simulink model "V2x_TC_TrafficLights_cs"  (Simulink 7.6)
settings: Solver=ode5  SolverMode=SingleTasking  StartTime=0.0  StopTime=38  FixedStep=1/100  RelTol=1e-3
PreLoadFcn:
  | check_NUTS_defined
  | 
  | assignin('base',['model_closed_' bdroot],false);addpath('../includes_V2x');
InitFcn:
  | PreScanExperimentInitFcn(bdroot)
StopFcn:
  | PreScanExperimentStopFcn(bdroot);
  | 
undefined symbols (not defined in the file or in its folder): acceleration, actorID, actorType, DiscIntNeverNeededParam, GPS_alt, GPS_lat, GPS_long, intmax, lightBarInUse, messageID, Newton, publicActorType, Raphson, rotZ, sigbuilder_block, sireneInUse, trafficLightPriority, turnAdvice, velocity, x_ini_VRML, y_ini_VRML, yawRate, z_ini_VRML
libraries: simulink x172, PreScanSimulinkLibrary x20, PreScanUsefulBlocks x11, simulink_extras x9, simulink_need_slupdate x1
(part 4 of 27)

== system V2x_TC_TrafficLights_cs/STATE_Sphere_1 ==
  State : Inport  SampleTime=TimeStep
  Acceleration : Subsystem  mask(ToSensorWorld){PortName=Acceleration_Sphere_1, PortIndex=1630, PortWidth=3, TimeStep=1/100}
      mask prompts: PortName "Port Name [-]"; PortIndex "Index [-]"; PortWidth "Port Dimensions [-]"; TimeStep "Upload Period [s]"
      mask help: Sends signal(s) from Simulink to the Sensor World
      mask Help:
      | Sends signal(s) from Simulink to the Sensor World.
      | 
      | - Port Name [-]: specifies the name of the output terminal of the Simulink block.
      | - Index [-]: specifies the start position in the output vector sent to the Sensor World
      | - Port dimensions [-]: specifies the width (number of scalars) of the output port
      | - Upload period [s]: specifies the upload period (in seconds) of the port.
      mask Display: port_label('input', 1, PortName)
  Demux : Demux  Outputs=5
  Position : Subsystem  mask(ToSensorWorld){PortName=Position_Sphere_1, PortIndex=1624, PortWidth=3, TimeStep=1/100}
      mask prompts: PortName "Port Name [-]"; PortIndex "Index [-]"; PortWidth "Port Dimensions [-]"; TimeStep "Upload Period [s]"
      mask help: Sends signal(s) from Simulink to the Sensor World
      mask Help:
      | Sends signal(s) from Simulink to the Sensor World.
      | 
      | - Port Name [-]: specifies the name of the output terminal of the Simulink block.
      | - Index [-]: specifies the start position in the output vector sent to the Sensor World
      | - Port dimensions [-]: specifies the width (number of scalars) of the output port
      | - Upload period [s]: specifies the upload period (in seconds) of the port.
      mask Display: port_label('input', 1, PortName)
  RPY : Subsystem  mask(ToSensorWorld){PortName=RPY_Sphere_1, PortIndex=1633, PortWidth=3, TimeStep=1/100}
      mask prompts: PortName "Port Name [-]"; PortIndex "Index [-]"; PortWidth "Port Dimensions [-]"; TimeStep "Upload Period [s]"
      mask help: Sends signal(s) from Simulink to the Sensor World
      mask Help:
      | Sends signal(s) from Simulink to the Sensor World.
      | 
      | - Port Name [-]: specifies the name of the output terminal of the Simulink block.
      | - Index [-]: specifies the start position in the output vector sent to the Sensor World
      | - Port dimensions [-]: specifies the width (number of scalars) of the output port
      | - Upload period [s]: specifies the upload period (in seconds) of the port.
      mask Display: port_label('input', 1, PortName)
  Velocity : Subsystem  mask(ToSensorWorld){PortName=Velocity_Sphere_1, PortIndex=1627, PortWidth=3, TimeStep=1/100}
      mask prompts: PortName "Port Name [-]"; PortIndex "Index [-]"; PortWidth "Port Dimensions [-]"; TimeStep "Upload Period [s]"
      mask help: Sends signal(s) from Simulink to the Sensor World
      mask Help:
      | Sends signal(s) from Simulink to the Sensor World.
      | 
      | - Port Name [-]: specifies the name of the output terminal of the Simulink block.
      | - Index [-]: specifies the start position in the output vector sent to the Sensor World
      | - Port dimensions [-]: specifies the width (number of scalars) of the output port
      | - Upload period [s]: specifies the upload period (in seconds) of the port.
      mask Display: port_label('input', 1, PortName)
  iniBODYAngles wrtVEH [rad] : Constant  Value=[Roll0 Pitch0 0]  SampleTime=TimeStep
  iniVEHAngles wrtGLOB [rad] : Constant  Value=[0 0 Yaw0]  SampleTime=TimeStep
  iniVEHposition wrtGLOB [m] : Constant  Value=[x0 y0 z0]  SampleTime=TimeStep
  state2FullState : PreScanSimulinkLibrary/state2FullState  SystemSampleTime=-1
  velRPY : Subsystem  mask(ToSensorWorld){PortName=velRPY_Sphere_1, PortIndex=1636, PortWidth=3, TimeStep=1/100}
      mask prompts: PortName "Port Name [-]"; PortIndex "Index [-]"; PortWidth "Port Dimensions [-]"; TimeStep "Upload Period [s]"
      mask help: Sends signal(s) from Simulink to the Sensor World
      mask Help:
      | Sends signal(s) from Simulink to the Sensor World.
      | 
      | - Port Name [-]: specifies the name of the output terminal of the Simulink block.
      | - Index [-]: specifies the start position in the output vector sent to the Sensor World
      | - Port dimensions [-]: specifies the width (number of scalars) of the output port
      | - Upload period [s]: specifies the upload period (in seconds) of the port.
      mask Display: port_label('input', 1, PortName)
  wires:
  Demux/5 -> velRPY/1(Y)
  Demux/4 -> RPY/1(Y)
  Demux/3 -> Acceleration/1(Y)
  Demux/2 -> Velocity/1(Y)
  Demux/1 -> Position/1(Y)
  State/1 -> state2FullState/1
  state2FullState/1 -> Demux/1
  iniVEHposition wrtGLOB [m]/1 -> state2FullState/2
  iniBODYAngles wrtVEH [rad]/1 -> state2FullState/3
  iniVEHAngles wrtGLOB [rad]/1 -> state2FullState/4

== system V2x_TC_TrafficLights_cs/SetActuators ==
  Bus Creator : BusCreator  Inputs=1
  Data Store Read : DataStoreRead  DataStoreName=SimCoreActuators  SampleTime=TimeStep
  SendActuators : M-S-Function  FunctionName=SendActuatorsBlock  Parameters=TimeStep NrOfActuators
  dummy : Outport
  tag scope: SimCoreInfo=global
  wires:
  Bus Creator/1 -> [SimCoreInfo]  "SimCore"
  SendActuators/2 -> Bus Creator/1  "ComputationTime[s]"
  SendActuators/1 -> dummy/1
  Data Store Read/1 -> SendActuators/1

== system V2x_TC_TrafficLights_cs/SimCoreInfo ==
  Bus Selector : BusSelector  OutputSignals=ComputationTime[s]
  Time : Scope
      settings: TimeRange=10  YMin=0  YMax=0.5  SaveName=ComputationTime
  wires:
  Bus Selector/1 -> Time/1  "<ComputationTime[s]>"
  [SimCoreInfo] -> Bus Selector/1

== system V2x_TC_TrafficLights_cs/Sphere_1 ==
  SELF_Sphere_1 : Inport  Tag=SELFInport
  Dynamics_Empty : Subsystem  Tag=Dynamics_Empty
      mask Display: port_label('output', 1, 'State');text(0,0,' Empty\n')
  Gain : Gain  Gain=[1 1 1 0 0 0 0 0 1]
  SELF_Demux : Subsystem  Tag=DemuxSELF
      settings: DeleteFcn=PreScanBlockDeleteFcn;
      mask Type: PreScan Demux Block:
      mask Display:
      | port_label('output',1,'x [m]')
      | port_label('output',2,'y [m]')
      | port_label('output',3,'z [m]','texmode','on')
      | port_label('output',4,'Rotx [deg]','texmode','on')
      | port_label('output',5,'Roty [deg]','texmode','on')
      | port_label('output',6,'Rotz [deg]','texmode','on')
      | port_label('output',7,'GPS lat [deg]','texmode','on')
      | port_label('output',8,'GPS long [deg]','texmode','on')
      | port_label('output',9,'GPS alt [m]','texmode','on')
      | port_label('output',10,'Velocity [m/s]')
      | port_label('output',11,'Heading [deg]')
      | port_label('output',12,'Yaw rate [deg/s]')
  Terminator_{1..13} (13 alike) : Terminator
  STATE_Sphere_1 : Outport  Tag=StateOutport
  wires:
  [State] -> Gain/1
  SELF_Sphere_1/1 -> SELF_Demux/1(SELF)
  Dynamics_Empty/1(State) -> Terminator_13/1
  Gain/1 -> STATE_Sphere_1/1
  SELF_Demux/1(x [m]) -> Terminator_1/1
  SELF_Demux/2(y [m]) -> Terminator_2/1
  SELF_Demux/3(z [m]) -> Terminator_3/1
  SELF_Demux/4(rotx [deg]) -> Terminator_4/1
  SELF_Demux/5(roty [deg]) -> Terminator_5/1
  SELF_Demux/6(rotz [deg]) -> Terminator_6/1
  SELF_Demux/7(GPS lat [deg]) -> Terminator_7/1
  SELF_Demux/8(GPS long [deg]) -> Terminator_8/1
  SELF_Demux/9(GPS alt [m]) -> Terminator_9/1
  SELF_Demux/10(velocity [m/s]) -> Terminator_10/1
  SELF_Demux/11(heading [deg]) -> Terminator_11/1
  SELF_Demux/12(yaw rate [deg/s]) -> Terminator_12/1

== system V2x_TC_TrafficLights_cs/TrafficLightRoadSideNL_1 ==
  Antenna_Tx_3_BusAssignment : BusAssignment  Tag=BusAntennaT  AssignedSignals=warning,messageID,actorID,actorType,trafficLightPriority,GPS_lat,GPS_long,GPS_alt,rotZ
  Antenna_Tx_3_Mux : Subsystem  Tag=MuxAntennaT  mask(PreScan Mux Block:){Channel=1, TimeStep=1/100}
      mask prompts: TimeStep "Time Step [s]"
      settings: DeleteFcn=PreScanBlockDeleteFcn;
      mask Display:
      | fprintf('Channel: %d',Channel)
      | port_label('input', 1, 'Warning signal ID')
      | port_label('input', 2, 'Maneuvre ID')
      | port_label('input', 3, 'Vehicle Type')
      | port_label('input', 4, 'Braking Capacity')
      | port_label('input', 5, 'Vehicle Size')
      | port_label('input', 6, 'spare')
  CAM generation time step : Constant  Value=0.1
  CAM repetition time step : Constant  Value=0.05
  Cartesian to GPS : Subsystem
  Ground_{1..5} (5 alike) : Ground
  Infrastructure CAM generator : Subsystem
  VISUCasingLights_Mux : Subsystem  Tag=MuxCasingLights
      settings: DeleteFcn=PreScanBlockDeleteFcn;
      mask Type: PreScan Mux Block:
  X : Constant  Value=112.02973175048828
  Y : Constant  Value=9.0402154922485352
  Z : Constant  Value=0
  actorID : Constant  Value=13
  actorType : Constant  Value=15
  different coordinate system conversion : Bias  Bias=-90
  lights : Subsystem
  messageID : Constant  Value=900
  rotZ : Constant  Value=270
  warning : Constant  Value=0
  Antenna_Tx_3 : Outport  Tag=AntennaTOutport
  VISUCasingLights_TrafficLightRoadSideNL_1 : Outport  Tag=VisuCasingLightsOutport  Port=2  SampleTime=1/100
  wires:
  CAM generation time step/1 -> Infrastructure CAM generator/1(CAM gen time step)
  CAM repetition time step/1 -> Infrastructure CAM generator/2(CAM rep time step)
  warning/1 -> Infrastructure CAM generator/3(warning)
  messageID/1 -> Infrastructure CAM generator/4(messageID)
  actorID/1 -> Infrastructure CAM generator/5(actorID)
  actorType/1 -> Infrastructure CAM generator/6(actorType)
  lights/1(R) -> Infrastructure CAM generator/7(Red light), VISUCasingLights_Mux/1(Red [-])
  lights/2(O) -> Infrastructure CAM generator/8(Orange light), VISUCasingLights_Mux/2(Orange [-])
  lights/3(G) -> Infrastructure CAM generator/9(Green light), VISUCasingLights_Mux/3(Green [-])
  rotZ/1 -> different coordinate system conversion/1
  Cartesian to GPS/1(lat) -> Infrastructure CAM generator/10(GPS_lat)
  Cartesian to GPS/2(long) -> Infrastructure CAM generator/11(GPS_long)
  Cartesian to GPS/3(alt) -> Infrastructure CAM generator/12(GPS_alt)
  X/1 -> Cartesian to GPS/1(X)
  Y/1 -> Cartesian to GPS/2(Y)
  Z/1 -> Cartesian to GPS/3(Z)
  different coordinate system conversion/1 -> Infrastructure CAM generator/13(rotZ)
  Antenna_Tx_3_Mux/2(Out2) -> [Antenna_Tx_3]
  [Antenna_Tx_3] -> Antenna_Tx_3_BusAssignment/1
  Antenna_Tx_3_BusAssignment/1 -> Antenna_Tx_3_Mux/6(spare)
  Antenna_Tx_3_Mux/1(Out1) -> Antenna_Tx_3/1
  VISUCasingLights_Mux/1(CasingLights) -> VISUCasingLights_TrafficLightRoadSideNL_1/1
  Infrastructure CAM generator/9(CAM_rotZ) -> Antenna_Tx_3_BusAssignment/10
  Infrastructure CAM generator/8(CAM_GPSalt) -> Antenna_Tx_3_BusAssignment/9
  Infrastructure CAM generator/7(CAM_GPSlong) -> Antenna_Tx_3_BusAssignment/8
  Infrastructure CAM generator/6(CAM_GPSlat) -> Antenna_Tx_3_BusAssignment/7
  Infrastructure CAM generator/5(CAM_trafficLightPriority) -> Antenna_Tx_3_BusAssignment/6
  Infrastructure CAM generator/4(CAM_vehicleType) -> Antenna_Tx_3_BusAssignment/5
  Infrastructure CAM generator/3(CAM_vehicleID) -> Antenna_Tx_3_BusAssignment/4
  Infrastructure CAM generator/2(CAM_messageID) -> Antenna_Tx_3_BusAssignment/3
  Infrastructure CAM generator/1(CAM_warning) -> Antenna_Tx_3_BusAssignment/2
  Ground_1/1 -> Antenna_Tx_3_Mux/1(warning_signal_id)
  Ground_2/1 -> Antenna_Tx_3_Mux/2(maneuvre_id)
  Ground_3/1 -> Antenna_Tx_3_Mux/3(vehicle_type)
  Ground_4/1 -> Antenna_Tx_3_Mux/4(brakingcapacity)
  Ground_5/1 -> Antenna_Tx_3_Mux/5(vehicle_size)

== system V2x_TC_TrafficLights_cs/TrafficLightRoadSideNL_2 ==
  Antenna_Tx_5_BusAssignment : BusAssignment  Tag=BusAntennaT  AssignedSignals=warning,messageID,actorID,actorType,trafficLightPriority,GPS_lat,GPS_long,GPS_alt,rotZ
  Antenna_Tx_5_Mux : Subsystem  Tag=MuxAntennaT  mask(PreScan Mux Block:){Channel=1, TimeStep=1/100}
      mask prompts: TimeStep "Time Step [s]"
      settings: DeleteFcn=PreScanBlockDeleteFcn;
      mask Display:
      | fprintf('Channel: %d',Channel)
      | port_label('input', 1, 'Warning signal ID')
      | port_label('input', 2, 'Maneuvre ID')
      | port_label('input', 3, 'Vehicle Type')
      | port_label('input', 4, 'Braking Capacity')
      | port_label('input', 5, 'Vehicle Size')
      | port_label('input', 6, 'spare')
  CAM generation time step : Constant  Value=0.1
  CAM repetition time step : Constant  Value=0.05
  Cartesian to GPS : Subsystem
  Ground_{1..5} (5 alike) : Ground
  Infrastructure CAM generator : Subsystem
  VISUCasingLights_Mux : Subsystem  Tag=MuxCasingLights
      settings: DeleteFcn=PreScanBlockDeleteFcn;
      mask Type: PreScan Mux Block:
  X : Constant  Value=123.47191619873047
  Y : Constant  Value=17.9848575592041
  Z : Constant  Value=0
  actorID : Constant  Value=16
  actorType : Constant  Value=15
  different coordinate system conversion : Bias  Bias=-90
  lights : Subsystem
  messageID : Constant  Value=900
  rotZ : Constant  Value=90
  warning : Constant  Value=0
  Antenna_Tx_5 : Outport  Tag=AntennaTOutport
  VISUCasingLights_TrafficLightRoadSideNL_2 : Outport  Tag=VisuCasingLightsOutport  Port=2  SampleTime=1/100
  wires:
  CAM generation time step/1 -> Infrastructure CAM generator/1(CAM gen time step)
  CAM repetition time step/1 -> Infrastructure CAM generator/2(CAM rep time step)
  warning/1 -> Infrastructure CAM generator/3(warning)
  messageID/1 -> Infrastructure CAM generator/4(messageID)
  actorID/1 -> Infrastructure CAM generator/5(actorID)
  actorType/1 -> Infrastructure CAM generator/6(actorType)
  lights/1(R) -> Infrastructure CAM generator/7(Red light), VISUCasingLights_Mux/1(Red [-])
  lights/2(O) -> Infrastructure CAM generator/8(Orange light), VISUCasingLights_Mux/2(Orange [-])
  lights/3(G) -> Infrastructure CAM generator/9(Green light), VISUCasingLights_Mux/3(Green [-])
  rotZ/1 -> different coordinate system conversion/1
  Cartesian to GPS/1(lat) -> Infrastructure CAM generator/10(GPS_lat)
  Cartesian to GPS/2(long) -> Infrastructure CAM generator/11(GPS_long)
  Cartesian to GPS/3(alt) -> Infrastructure CAM generator/12(GPS_alt)
  X/1 -> Cartesian to GPS/1(X)
  Y/1 -> Cartesian to GPS/2(Y)
  Z/1 -> Cartesian to GPS/3(Z)
  different coordinate system conversion/1 -> Infrastructure CAM generator/13(rotZ)
  Antenna_Tx_5_Mux/2(Out2) -> [Antenna_Tx_5]
  [Antenna_Tx_5] -> Antenna_Tx_5_BusAssignment/1
  Antenna_Tx_5_BusAssignment/1 -> Antenna_Tx_5_Mux/6(spare)
  Antenna_Tx_5_Mux/1(Out1) -> Antenna_Tx_5/1
  VISUCasingLights_Mux/1(CasingLights) -> VISUCasingLights_TrafficLightRoadSideNL_2/1
  Infrastructure CAM generator/9(CAM_rotZ) -> Antenna_Tx_5_BusAssignment/10
  Infrastructure CAM generator/8(CAM_GPSalt) -> Antenna_Tx_5_BusAssignment/9
  Infrastructure CAM generator/7(CAM_GPSlong) -> Antenna_Tx_5_BusAssignment/8
  Infrastructure CAM generator/6(CAM_GPSlat) -> Antenna_Tx_5_BusAssignment/7
  Infrastructure CAM generator/5(CAM_trafficLightPriority) -> Antenna_Tx_5_BusAssignment/6
  Infrastructure CAM generator/4(CAM_vehicleType) -> Antenna_Tx_5_BusAssignment/5
  Infrastructure CAM generator/3(CAM_vehicleID) -> Antenna_Tx_5_BusAssignment/4
  Infrastructure CAM generator/2(CAM_messageID) -> Antenna_Tx_5_BusAssignment/3
  Infrastructure CAM generator/1(CAM_warning) -> Antenna_Tx_5_BusAssignment/2
  Ground_1/1 -> Antenna_Tx_5_Mux/1(warning_signal_id)
  Ground_2/1 -> Antenna_Tx_5_Mux/2(maneuvre_id)
  Ground_3/1 -> Antenna_Tx_5_Mux/3(vehicle_type)
  Ground_4/1 -> Antenna_Tx_5_Mux/4(brakingcapacity)
  Ground_5/1 -> Antenna_Tx_5_Mux/5(vehicle_size)

== system V2x_TC_TrafficLights_cs/TrafficLightRoadSideNL_3 ==
  Antenna_Tx_6_BusAssignment : BusAssignment  Tag=BusAntennaT  AssignedSignals=warning,messageID,actorID,actorType,trafficLightPriority,GPS_lat,GPS_long,GPS_alt,rotZ
  Antenna_Tx_6_Mux : Subsystem  Tag=MuxAntennaT  mask(PreScan Mux Block:){Channel=1, TimeStep=1/100}
      mask prompts: TimeStep "Time Step [s]"
      settings: DeleteFcn=PreScanBlockDeleteFcn;
      mask Display:
      | fprintf('Channel: %d',Channel)
      | port_label('input', 1, 'Warning signal ID')
      | port_label('input', 2, 'Maneuvre ID')
      | port_label('input', 3, 'Vehicle Type')
      | port_label('input', 4, 'Braking Capacity')
      | port_label('input', 5, 'Vehicle Size')
      | port_label('input', 6, 'spare')
  CAM generation time step : Constant  Value=0.1
  CAM repetition time step : Constant  Value=0.05
  Cartesian to GPS : Subsystem
  Ground_{1..5} (5 alike) : Ground
  Infrastructure CAM generator : Subsystem
  VISUCasingLights_Mux : Subsystem  Tag=MuxCasingLights
      settings: DeleteFcn=PreScanBlockDeleteFcn;
      mask Type: PreScan Mux Block:
  X : Constant  Value=122.22503662109375
  Y : Constant  Value=7.7345576286315918
  Z : Constant  Value=0
  actorID : Constant  Value=14
  actorType : Constant  Value=15
  different coordinate system conversion : Bias  Bias=-90
  lights : Subsystem
  messageID : Constant  Value=900
  rotZ : Constant  Value=0
  warning : Constant  Value=0
  Antenna_Tx_6 : Outport  Tag=AntennaTOutport
  VISUCasingLights_TrafficLightRoadSideNL_3 : Outport  Tag=VisuCasingLightsOutport  Port=2  SampleTime=1/100
  wires:
  CAM generation time step/1 -> Infrastructure CAM generator/1(CAM gen time step)
  CAM repetition time step/1 -> Infrastructure CAM generator/2(CAM rep time step)
  warning/1 -> Infrastructure CAM generator/3(warning)
  messageID/1 -> Infrastructure CAM generator/4(messageID)
  actorID/1 -> Infrastructure CAM generator/5(actorID)
  actorType/1 -> Infrastructure CAM generator/6(actorType)
  rotZ/1 -> different coordinate system conversion/1
  Cartesian to GPS/1(lat) -> Infrastructure CAM generator/10(GPS_lat)
  Cartesian to GPS/2(long) -> Infrastructure CAM generator/11(GPS_long)
  Cartesian to GPS/3(alt) -> Infrastructure CAM generator/12(GPS_alt)
  X/1 -> Cartesian to GPS/1(X)
  Y/1 -> Cartesian to GPS/2(Y)
  Z/1 -> Cartesian to GPS/3(Z)
  lights/1(R) -> Infrastructure CAM generator/7(Red light), VISUCasingLights_Mux/1(Red [-])
  lights/2(O) -> Infrastructure CAM generator/8(Orange light), VISUCasingLights_Mux/2(Orange [-])
  lights/3(G) -> Infrastructure CAM generator/9(Green light), VISUCasingLights_Mux/3(Green [-])
  different coordinate system conversion/1 -> Infrastructure CAM generator/13(rotZ)
  Antenna_Tx_6_Mux/2(Out2) -> [Antenna_Tx_6]
  [Antenna_Tx_6] -> Antenna_Tx_6_BusAssignment/1
  Antenna_Tx_6_BusAssignment/1 -> Antenna_Tx_6_Mux/6(spare)
  Antenna_Tx_6_Mux/1(Out1) -> Antenna_Tx_6/1
  VISUCasingLights_Mux/1(CasingLights) -> VISUCasingLights_TrafficLightRoadSideNL_3/1
  Infrastructure CAM generator/9(CAM_rotZ) -> Antenna_Tx_6_BusAssignment/10
  Infrastructure CAM generator/8(CAM_GPSalt) -> Antenna_Tx_6_BusAssignment/9
  Infrastructure CAM generator/7(CAM_GPSlong) -> Antenna_Tx_6_BusAssignment/8
  Infrastructure CAM generator/6(CAM_GPSlat) -> Antenna_Tx_6_BusAssignment/7
  Infrastructure CAM generator/5(CAM_trafficLightPriority) -> Antenna_Tx_6_BusAssignment/6
  Infrastructure CAM generator/4(CAM_vehicleType) -> Antenna_Tx_6_BusAssignment/5
  Infrastructure CAM generator/3(CAM_vehicleID) -> Antenna_Tx_6_BusAssignment/4
  Infrastructure CAM generator/2(CAM_messageID) -> Antenna_Tx_6_BusAssignment/3
  Infrastructure CAM generator/1(CAM_warning) -> Antenna_Tx_6_BusAssignment/2
  Ground_1/1 -> Antenna_Tx_6_Mux/1(warning_signal_id)
  Ground_2/1 -> Antenna_Tx_6_Mux/2(maneuvre_id)
  Ground_3/1 -> Antenna_Tx_6_Mux/3(vehicle_type)
  Ground_4/1 -> Antenna_Tx_6_Mux/4(brakingcapacity)
  Ground_5/1 -> Antenna_Tx_6_Mux/5(vehicle_size)

== system V2x_TC_TrafficLights_cs/TrafficLightRoadSideNL_4 ==
  Antenna_Tx_4_BusAssignment : BusAssignment  Tag=BusAntennaT  AssignedSignals=warning,messageID,actorID,actorType,trafficLightPriority,GPS_lat,GPS_long,GPS_alt,rotZ
  Antenna_Tx_4_Mux : Subsystem  Tag=MuxAntennaT  mask(PreScan Mux Block:){Channel=1, TimeStep=1/100}
      mask prompts: TimeStep "Time Step [s]"
      settings: DeleteFcn=PreScanBlockDeleteFcn;
      mask Display:
      | fprintf('Channel: %d',Channel)
      | port_label('input', 1, 'Warning signal ID')
      | port_label('input', 2, 'Maneuvre ID')
      | port_label('input', 3, 'Vehicle Type')
      | port_label('input', 4, 'Braking Capacity')
      | port_label('input', 5, 'Vehicle Size')
      | port_label('input', 6, 'spare')
  CAM generation time step : Constant  Value=0.1
  CAM repetition time step : Constant  Value=0.05
  Cartesian to GPS : Subsystem
  Ground_{1..5} (5 alike) : Ground
  Infrastructure CAM generator : Subsystem
  VISUCasingLights_Mux : Subsystem  Tag=MuxCasingLights
      settings: DeleteFcn=PreScanBlockDeleteFcn;
      mask Type: PreScan Mux Block:
  X : Constant  Value=113.2569580078125
  Y : Constant  Value=19.242471694946289
  Z : Constant  Value=0
  actorID : Constant  Value=17
  actorType : Constant  Value=15
  different coordinate system conversion : Bias  Bias=-90
  lights : Subsystem
  messageID : Constant  Value=900
  rotZ : Constant  Value=180
  warning : Constant  Value=0
  Antenna_Tx_4 : Outport  Tag=AntennaTOutport
  VISUCasingLights_TrafficLightRoadSideNL_4 : Outport  Tag=VisuCasingLightsOutport  Port=2  SampleTime=1/100
  wires:
  CAM generation time step/1 -> Infrastructure CAM generator/1(CAM gen time step)
  CAM repetition time step/1 -> Infrastructure CAM generator/2(CAM rep time step)
  warning/1 -> Infrastructure CAM generator/3(warning)
  messageID/1 -> Infrastructure CAM generator/4(messageID)
  actorID/1 -> Infrastructure CAM generator/5(actorID)
  actorType/1 -> Infrastructure CAM generator/6(actorType)
  rotZ/1 -> different coordinate system conversion/1
  Cartesian to GPS/1(lat) -> Infrastructure CAM generator/10(GPS_lat)
  Cartesian to GPS/2(long) -> Infrastructure CAM generator/11(GPS_long)
  Cartesian to GPS/3(alt) -> Infrastructure CAM generator/12(GPS_alt)
  X/1 -> Cartesian to GPS/1(X)
  Y/1 -> Cartesian to GPS/2(Y)
  Z/1 -> Cartesian to GPS/3(Z)
  lights/1(R) -> Infrastructure CAM generator/7(Red light), VISUCasingLights_Mux/1(Red [-])
  lights/2(O) -> Infrastructure CAM generator/8(Orange light), VISUCasingLights_Mux/2(Orange [-])
  lights/3(G) -> Infrastructure CAM generator/9(Green light), VISUCasingLights_Mux/3(Green [-])
  different coordinate system conversion/1 -> Infrastructure CAM generator/13(rotZ)
  Antenna_Tx_4_Mux/2(Out2) -> [Antenna_Tx_4]
  [Antenna_Tx_4] -> Antenna_Tx_4_BusAssignment/1
  Antenna_Tx_4_BusAssignment/1 -> Antenna_Tx_4_Mux/6(spare)
  Antenna_Tx_4_Mux/1(Out1) -> Antenna_Tx_4/1
  VISUCasingLights_Mux/1(CasingLights) -> VISUCasingLights_TrafficLightRoadSideNL_4/1
  Infrastructure CAM generator/9(CAM_rotZ) -> Antenna_Tx_4_BusAssignment/10
  Infrastructure CAM generator/8(CAM_GPSalt) -> Antenna_Tx_4_BusAssignment/9
  Infrastructure CAM generator/7(CAM_GPSlong) -> Antenna_Tx_4_BusAssignment/8
  Infrastructure CAM generator/6(CAM_GPSlat) -> Antenna_Tx_4_BusAssignment/7
  Infrastructure CAM generator/5(CAM_trafficLightPriority) -> Antenna_Tx_4_BusAssignment/6
  Infrastructure CAM generator/4(CAM_vehicleType) -> Antenna_Tx_4_BusAssignment/5
  Infrastructure CAM generator/3(CAM_vehicleID) -> Antenna_Tx_4_BusAssignment/4
  Infrastructure CAM generator/2(CAM_messageID) -> Antenna_Tx_4_BusAssignment/3
  Infrastructure CAM generator/1(CAM_warning) -> Antenna_Tx_4_BusAssignment/2
  Ground_1/1 -> Antenna_Tx_4_Mux/1(warning_signal_id)
  Ground_2/1 -> Antenna_Tx_4_Mux/2(maneuvre_id)
  Ground_3/1 -> Antenna_Tx_4_Mux/3(vehicle_type)
  Ground_4/1 -> Antenna_Tx_4_Mux/4(brakingcapacity)
  Ground_5/1 -> Antenna_Tx_4_Mux/5(vehicle_size)

== system V2x_TC_TrafficLights_cs/TrafficLightRoadSideNL_5 ==
  Antenna_Tx_16_BusAssignment : BusAssignment  Tag=BusAntennaT  AssignedSignals=warning,messageID,actorID,actorType,trafficLightPriority,GPS_lat,GPS_long,GPS_alt,rotZ
  Antenna_Tx_16_Mux : Subsystem  Tag=MuxAntennaT  mask(PreScan Mux Block:){Channel=1, TimeStep=1/100}
      mask prompts: TimeStep "Time Step [s]"
      settings: DeleteFcn=PreScanBlockDeleteFcn;
      mask Display:
      | fprintf('Channel: %d',Channel)
      | port_label('input', 1, 'Warning signal ID')
      | port_label('input', 2, 'Maneuvre ID')
      | port_label('input', 3, 'Vehicle Type')
      | port_label('input', 4, 'Braking Capacity')
      | port_label('input', 5, 'Vehicle Size')
      | port_label('input', 6, 'spare')
  CAM generation time step : Constant  Value=0.1
  CAM repetition time step : Constant  Value=0.05
  Cartesian to GPS : Subsystem
  Ground_{1..5} (5 alike) : Ground
  Infrastructure CAM generator : Subsystem
  VISUCasingLights_Mux : Subsystem  Tag=MuxCasingLights
      settings: DeleteFcn=PreScanBlockDeleteFcn;
      mask Type: PreScan Mux Block:
  X : Constant  Value=180.04579162597656
  Y : Constant  Value=76.942386627197266
  Z : Constant  Value=0
  actorID : Constant  Value=19
  actorType : Constant  Value=15
  different coordinate system conversion : Bias  Bias=-90
  lights : Subsystem
  messageID : Constant  Value=900
  rotZ : Constant  Value=270
  warning : Constant  Value=0
  Antenna_Tx_16 : Outport  Tag=AntennaTOutport
  VISUCasingLights_TrafficLightRoadSideNL_5 : Outport  Tag=VisuCasingLightsOutport  Port=2  SampleTime=1/100
  wires:
  CAM generation time step/1 -> Infrastructure CAM generator/1(CAM gen time step)
  CAM repetition time step/1 -> Infrastructure CAM generator/2(CAM rep time step)
  warning/1 -> Infrastructure CAM generator/3(warning)
  messageID/1 -> Infrastructure CAM generator/4(messageID)
  actorID/1 -> Infrastructure CAM generator/5(actorID)
  actorType/1 -> Infrastructure CAM generator/6(actorType)
  rotZ/1 -> different coordinate system conversion/1
  Cartesian to GPS/1(lat) -> Infrastructure CAM generator/10(GPS_lat)
  Cartesian to GPS/2(long) -> Infrastructure CAM generator/11(GPS_long)
  Cartesian to GPS/3(alt) -> Infrastructure CAM generator/12(GPS_alt)
  X/1 -> Cartesian to GPS/1(X)
  Y/1 -> Cartesian to GPS/2(Y)
  Z/1 -> Cartesian to GPS/3(Z)
  lights/1(R) -> Infrastructure CAM generator/7(Red light), VISUCasingLights_Mux/1(Red [-])
  lights/2(O) -> Infrastructure CAM generator/8(Orange light), VISUCasingLights_Mux/2(Orange [-])
  lights/3(G) -> Infrastructure CAM generator/9(Green light), VISUCasingLights_Mux/3(Green [-])
  different coordinate system conversion/1 -> Infrastructure CAM generator/13(rotZ)
  Antenna_Tx_16_Mux/2(Out2) -> [Antenna_Tx_16]
  [Antenna_Tx_16] -> Antenna_Tx_16_BusAssignment/1
  Antenna_Tx_16_BusAssignment/1 -> Antenna_Tx_16_Mux/6(spare)
  Antenna_Tx_16_Mux/1(Out1) -> Antenna_Tx_16/1
  VISUCasingLights_Mux/1(CasingLights) -> VISUCasingLights_TrafficLightRoadSideNL_5/1
  Infrastructure CAM generator/9(CAM_rotZ) -> Antenna_Tx_16_BusAssignment/10
  Infrastructure CAM generator/8(CAM_GPSalt) -> Antenna_Tx_16_BusAssignment/9
  Infrastructure CAM generator/7(CAM_GPSlong) -> Antenna_Tx_16_BusAssignment/8
  Infrastructure CAM generator/6(CAM_GPSlat) -> Antenna_Tx_16_BusAssignment/7
  Infrastructure CAM generator/5(CAM_trafficLightPriority) -> Antenna_Tx_16_BusAssignment/6
  Infrastructure CAM generator/4(CAM_vehicleType) -> Antenna_Tx_16_BusAssignment/5
  Infrastructure CAM generator/3(CAM_vehicleID) -> Antenna_Tx_16_BusAssignment/4
  Infrastructure CAM generator/2(CAM_messageID) -> Antenna_Tx_16_BusAssignment/3
  Infrastructure CAM generator/1(CAM_warning) -> Antenna_Tx_16_BusAssignment/2
  Ground_1/1 -> Antenna_Tx_16_Mux/1(warning_signal_id)
  Ground_2/1 -> Antenna_Tx_16_Mux/2(maneuvre_id)
  Ground_3/1 -> Antenna_Tx_16_Mux/3(vehicle_type)
  Ground_4/1 -> Antenna_Tx_16_Mux/4(brakingcapacity)
  Ground_5/1 -> Antenna_Tx_16_Mux/5(vehicle_size)

== system V2x_TC_TrafficLights_cs/TrafficLightRoadSideNL_6 ==
  Antenna_Tx_17_BusAssignment : BusAssignment  Tag=BusAntennaT  AssignedSignals=warning,messageID,actorID,actorType,trafficLightPriority,GPS_lat,GPS_long,GPS_alt,rotZ
  Antenna_Tx_17_Mux : Subsystem  Tag=MuxAntennaT  mask(PreScan Mux Block:){Channel=1, TimeStep=1/100}
      mask prompts: TimeStep "Time Step [s]"
      settings: DeleteFcn=PreScanBlockDeleteFcn;
      mask Display:
      | fprintf('Channel: %d',Channel)
      | port_label('input', 1, 'Warning signal ID')
      | port_label('input', 2, 'Maneuvre ID')
      | port_label('input', 3, 'Vehicle Type')
      | port_label('input', 4, 'Braking Capacity')
      | port_label('input', 5, 'Vehicle Size')
      | port_label('input', 6, 'spare')
  CAM generation time step : Constant  Value=0.1
  CAM repetition time step : Constant  Value=0.05
  Cartesian to GPS : Subsystem
  Ground_{1..5} (5 alike) : Ground
  Infrastructure CAM generator : Subsystem
  VISUCasingLights_Mux : Subsystem  Tag=MuxCasingLights
      settings: DeleteFcn=PreScanBlockDeleteFcn;
      mask Type: PreScan Mux Block:
  X : Constant  Value=191.30799102783203
  Y : Constant  Value=85.8631591796875
  Z : Constant  Value=0
  actorID : Constant  Value=20
  actorType : Constant  Value=15
  different coordinate system conversion : Bias  Bias=-90
  lights : Subsystem
  messageID : Constant  Value=900
  rotZ : Constant  Value=90
  warning : Constant  Value=0
  Antenna_Tx_17 : Outport  Tag=AntennaTOutport
  VISUCasingLights_TrafficLightRoadSideNL_6 : Outport  Tag=VisuCasingLightsOutport  Port=2  SampleTime=1/100
  wires:
  CAM generation time step/1 -> Infrastructure CAM generator/1(CAM gen time step)
  CAM repetition time step/1 -> Infrastructure CAM generator/2(CAM rep time step)
  warning/1 -> Infrastructure CAM generator/3(warning)
  messageID/1 -> Infrastructure CAM generator/4(messageID)
  actorID/1 -> Infrastructure CAM generator/5(actorID)
  actorType/1 -> Infrastructure CAM generator/6(actorType)
  rotZ/1 -> different coordinate system conversion/1
  Cartesian to GPS/1(lat) -> Infrastructure CAM generator/10(GPS_lat)
  Cartesian to GPS/2(long) -> Infrastructure CAM generator/11(GPS_long)
  Cartesian to GPS/3(alt) -> Infrastructure CAM generator/12(GPS_alt)
  X/1 -> Cartesian to GPS/1(X)
  Y/1 -> Cartesian to GPS/2(Y)
  Z/1 -> Cartesian to GPS/3(Z)
  lights/1(R) -> Infrastructure CAM generator/7(Red light), VISUCasingLights_Mux/1(Red [-])
  lights/2(O) -> Infrastructure CAM generator/8(Orange light), VISUCasingLights_Mux/2(Orange [-])
  lights/3(G) -> Infrastructure CAM generator/9(Green light), VISUCasingLights_Mux/3(Green [-])
  different coordinate system conversion/1 -> Infrastructure CAM generator/13(rotZ)
  Antenna_Tx_17_Mux/2(Out2) -> [Antenna_Tx_17]
  [Antenna_Tx_17] -> Antenna_Tx_17_BusAssignment/1
  Antenna_Tx_17_BusAssignment/1 -> Antenna_Tx_17_Mux/6(spare)
  Antenna_Tx_17_Mux/1(Out1) -> Antenna_Tx_17/1
  VISUCasingLights_Mux/1(CasingLights) -> VISUCasingLights_TrafficLightRoadSideNL_6/1
  Infrastructure CAM generator/9(CAM_rotZ) -> Antenna_Tx_17_BusAssignment/10
  Infrastructure CAM generator/8(CAM_GPSalt) -> Antenna_Tx_17_BusAssignment/9
  Infrastructure CAM generator/7(CAM_GPSlong) -> Antenna_Tx_17_BusAssignment/8
  Infrastructure CAM generator/6(CAM_GPSlat) -> Antenna_Tx_17_BusAssignment/7
  Infrastructure CAM generator/5(CAM_trafficLightPriority) -> Antenna_Tx_17_BusAssignment/6
  Infrastructure CAM generator/4(CAM_vehicleType) -> Antenna_Tx_17_BusAssignment/5
  Infrastructure CAM generator/3(CAM_vehicleID) -> Antenna_Tx_17_BusAssignment/4
  Infrastructure CAM generator/2(CAM_messageID) -> Antenna_Tx_17_BusAssignment/3
  Infrastructure CAM generator/1(CAM_warning) -> Antenna_Tx_17_BusAssignment/2
  Ground_1/1 -> Antenna_Tx_17_Mux/1(warning_signal_id)
  Ground_2/1 -> Antenna_Tx_17_Mux/2(maneuvre_id)
  Ground_3/1 -> Antenna_Tx_17_Mux/3(vehicle_type)
  Ground_4/1 -> Antenna_Tx_17_Mux/4(brakingcapacity)
  Ground_5/1 -> Antenna_Tx_17_Mux/5(vehicle_size)

== system V2x_TC_TrafficLights_cs/TrafficLightRoadSideNL_7 ==
  Antenna_Tx_18_BusAssignment : BusAssignment  Tag=BusAntennaT  AssignedSignals=warning,messageID,actorID,actorType,trafficLightPriority,GPS_lat,GPS_long,GPS_alt,rotZ
  Antenna_Tx_18_Mux : Subsystem  Tag=MuxAntennaT  mask(PreScan Mux Block:){Channel=1, TimeStep=1/100}
      mask prompts: TimeStep "Time Step [s]"
      settings: DeleteFcn=PreScanBlockDeleteFcn;
      mask Display:
      | fprintf('Channel: %d',Channel)
      | port_label('input', 1, 'Warning signal ID')
      | port_label('input', 2, 'Maneuvre ID')
      | port_label('input', 3, 'Vehicle Type')
      | port_label('input', 4, 'Braking Capacity')
      | port_label('input', 5, 'Vehicle Size')
      | port_label('input', 6, 'spare')
  CAM generation time step : Constant  Value=0.1
  CAM repetition time step : Constant  Value=0.05
  Cartesian to GPS : Subsystem
  Ground_{1..5} (5 alike) : Ground
  Infrastructure CAM generator : Subsystem
  VISUCasingLights_Mux : Subsystem  Tag=MuxCasingLights
      settings: DeleteFcn=PreScanBlockDeleteFcn;
      mask Type: PreScan Mux Block:
  X : Constant  Value=190.02272033691406
  Y : Constant  Value=75.821640968322754
  Z : Constant  Value=0
  actorID : Constant  Value=21
  actorType : Constant  Value=15
  different coordinate system conversion : Bias  Bias=-90
  lights : Subsystem
  messageID : Constant  Value=900
  rotZ : Constant  Value=0
  warning : Constant  Value=0
  Antenna_Tx_18 : Outport  Tag=AntennaTOutport
  VISUCasingLights_TrafficLightRoadSideNL_7 : Outport  Tag=VisuCasingLightsOutport  Port=2  SampleTime=1/100
  wires:
  CAM generation time step/1 -> Infrastructure CAM generator/1(CAM gen time step)
  CAM repetition time step/1 -> Infrastructure CAM generator/2(CAM rep time step)
  warning/1 -> Infrastructure CAM generator/3(warning)
  messageID/1 -> Infrastructure CAM generator/4(messageID)
  actorID/1 -> Infrastructure CAM generator/5(actorID)
  actorType/1 -> Infrastructure CAM generator/6(actorType)
  rotZ/1 -> different coordinate system conversion/1
  Cartesian to GPS/1(lat) -> Infrastructure CAM generator/10(GPS_lat)
  Cartesian to GPS/2(long) -> Infrastructure CAM generator/11(GPS_long)
  Cartesian to GPS/3(alt) -> Infrastructure CAM generator/12(GPS_alt)
  X/1 -> Cartesian to GPS/1(X)
  Y/1 -> Cartesian to GPS/2(Y)
  Z/1 -> Cartesian to GPS/3(Z)
  lights/1(R) -> Infrastructure CAM generator/7(Red light), VISUCasingLights_Mux/1(Red [-])
  lights/2(O) -> Infrastructure CAM generator/8(Orange light), VISUCasingLights_Mux/2(Orange [-])
  lights/3(G) -> Infrastructure CAM generator/9(Green light), VISUCasingLights_Mux/3(Green [-])
  different coordinate system conversion/1 -> Infrastructure CAM generator/13(rotZ)
  Antenna_Tx_18_Mux/2(Out2) -> [Antenna_Tx_18]
  [Antenna_Tx_18] -> Antenna_Tx_18_BusAssignment/1
  Antenna_Tx_18_BusAssignment/1 -> Antenna_Tx_18_Mux/6(spare)
  Antenna_Tx_18_Mux/1(Out1) -> Antenna_Tx_18/1
  VISUCasingLights_Mux/1(CasingLights) -> VISUCasingLights_TrafficLightRoadSideNL_7/1
  Infrastructure CAM generator/9(CAM_rotZ) -> Antenna_Tx_18_BusAssignment/10
  Infrastructure CAM generator/8(CAM_GPSalt) -> Antenna_Tx_18_BusAssignment/9
  Infrastructure CAM generator/7(CAM_GPSlong) -> Antenna_Tx_18_BusAssignment/8
  Infrastructure CAM generator/6(CAM_GPSlat) -> Antenna_Tx_18_BusAssignment/7
  Infrastructure CAM generator/5(CAM_trafficLightPriority) -> Antenna_Tx_18_BusAssignment/6
  Infrastructure CAM generator/4(CAM_vehicleType) -> Antenna_Tx_18_BusAssignment/5
  Infrastructure CAM generator/3(CAM_vehicleID) -> Antenna_Tx_18_BusAssignment/4
  Infrastructure CAM generator/2(CAM_messageID) -> Antenna_Tx_18_BusAssignment/3
  Infrastructure CAM generator/1(CAM_warning) -> Antenna_Tx_18_BusAssignment/2
  Ground_1/1 -> Antenna_Tx_18_Mux/1(warning_signal_id)
  Ground_2/1 -> Antenna_Tx_18_Mux/2(maneuvre_id)
  Ground_3/1 -> Antenna_Tx_18_Mux/3(vehicle_type)
  Ground_4/1 -> Antenna_Tx_18_Mux/4(brakingcapacity)
  Ground_5/1 -> Antenna_Tx_18_Mux/5(vehicle_size)

== system V2x_TC_TrafficLights_cs/TrafficLightRoadSideNL_8 ==
  Antenna_Tx_19_BusAssignment : BusAssignment  Tag=BusAntennaT  AssignedSignals=warning,messageID,actorID,actorType,trafficLightPriority,GPS_lat,GPS_long,GPS_alt,rotZ
  Antenna_Tx_19_Mux : Subsystem  Tag=MuxAntennaT  mask(PreScan Mux Block:){Channel=1, TimeStep=1/100}
      mask prompts: TimeStep "Time Step [s]"
      settings: DeleteFcn=PreScanBlockDeleteFcn;
      mask Display:
      | fprintf('Channel: %d',Channel)
      | port_label('input', 1, 'Warning signal ID')
      | port_label('input', 2, 'Maneuvre ID')
      | port_label('input', 3, 'Vehicle Type')
      | port_label('input', 4, 'Braking Capacity')
      | port_label('input', 5, 'Vehicle Size')
      | port_label('input', 6, 'spare')
  CAM generation time step : Constant  Value=0.1
  CAM repetition time step : Constant  Value=0.05
  Cartesian to GPS : Subsystem
  Ground_{1..5} (5 alike) : Ground
  Infrastructure CAM generator : Subsystem
  VISUCasingLights_Mux : Subsystem  Tag=MuxCasingLights
      settings: DeleteFcn=PreScanBlockDeleteFcn;
      mask Type: PreScan Mux Block:
  X : Constant  Value=181.20407104492188
  Y : Constant  Value=87.144790649414063
  Z : Constant  Value=0
  actorID : Constant  Value=22
  actorType : Constant  Value=15
  different coordinate system conversion : Bias  Bias=-90
  lights : Subsystem
  messageID : Constant  Value=900
  rotZ : Constant  Value=180
  warning : Constant  Value=0
  Antenna_Tx_19 : Outport  Tag=AntennaTOutport
  VISUCasingLights_TrafficLightRoadSideNL_8 : Outport  Tag=VisuCasingLightsOutport  Port=2  SampleTime=1/100
  wires:
  CAM generation time step/1 -> Infrastructure CAM generator/1(CAM gen time step)
  CAM repetition time step/1 -> Infrastructure CAM generator/2(CAM rep time step)
  warning/1 -> Infrastructure CAM generator/3(warning)
  messageID/1 -> Infrastructure CAM generator/4(messageID)
  actorID/1 -> Infrastructure CAM generator/5(actorID)
  actorType/1 -> Infrastructure CAM generator/6(actorType)
  rotZ/1 -> different coordinate system conversion/1
  Cartesian to GPS/1(lat) -> Infrastructure CAM generator/10(GPS_lat)
  Cartesian to GPS/2(long) -> Infrastructure CAM generator/11(GPS_long)
  Cartesian to GPS/3(alt) -> Infrastructure CAM generator/12(GPS_alt)
  X/1 -> Cartesian to GPS/1(X)
  Y/1 -> Cartesian to GPS/2(Y)
  Z/1 -> Cartesian to GPS/3(Z)
  lights/1(R) -> Infrastructure CAM generator/7(Red light), VISUCasingLights_Mux/1(Red [-])
  lights/2(O) -> Infrastructure CAM generator/8(Orange light), VISUCasingLights_Mux/2(Orange [-])
  lights/3(G) -> Infrastructure CAM generator/9(Green light), VISUCasingLights_Mux/3(Green [-])
  different coordinate system conversion/1 -> Infrastructure CAM generator/13(rotZ)
  Antenna_Tx_19_Mux/2(Out2) -> [Antenna_Tx_19]
  [Antenna_Tx_19] -> Antenna_Tx_19_BusAssignment/1
  Antenna_Tx_19_BusAssignment/1 -> Antenna_Tx_19_Mux/6(spare)
  Antenna_Tx_19_Mux/1(Out1) -> Antenna_Tx_19/1
  VISUCasingLights_Mux/1(CasingLights) -> VISUCasingLights_TrafficLightRoadSideNL_8/1
  Infrastructure CAM generator/9(CAM_rotZ) -> Antenna_Tx_19_BusAssignment/10
  Infrastructure CAM generator/8(CAM_GPSalt) -> Antenna_Tx_19_BusAssignment/9
  Infrastructure CAM generator/7(CAM_GPSlong) -> Antenna_Tx_19_BusAssignment/8
  Infrastructure CAM generator/6(CAM_GPSlat) -> Antenna_Tx_19_BusAssignment/7
  Infrastructure CAM generator/5(CAM_trafficLightPriority) -> Antenna_Tx_19_BusAssignment/6
  Infrastructure CAM generator/4(CAM_vehicleType) -> Antenna_Tx_19_BusAssignment/5
  Infrastructure CAM generator/3(CAM_vehicleID) -> Antenna_Tx_19_BusAssignment/4
  Infrastructure CAM generator/2(CAM_messageID) -> Antenna_Tx_19_BusAssignment/3
  Infrastructure CAM generator/1(CAM_warning) -> Antenna_Tx_19_BusAssignment/2
  Ground_1/1 -> Antenna_Tx_19_Mux/1(warning_signal_id)
  Ground_2/1 -> Antenna_Tx_19_Mux/2(maneuvre_id)
  Ground_3/1 -> Antenna_Tx_19_Mux/3(vehicle_type)
  Ground_4/1 -> Antenna_Tx_19_Mux/4(brakingcapacity)
  Ground_5/1 -> Antenna_Tx_19_Mux/5(vehicle_size)

== system V2x_TC_TrafficLights_cs/TrafficSignPoleNL_1 ==
  Antenna_Tx_8_BusAssignment : BusAssignment  Tag=BusAntennaT  AssignedSignals=warning,messageID,actorID,actorType,trafficLightPriority,GPS_lat,GPS_long,GPS_alt,rotZ
  Antenna_Tx_8_Mux : Subsystem  Tag=MuxAntennaT  mask(PreScan Mux Block:){Channel=1, TimeStep=1/100}
      mask prompts: TimeStep "Time Step [s]"
      settings: DeleteFcn=PreScanBlockDeleteFcn;
      mask Display:
      | fprintf('Channel: %d',Channel)
      | port_label('input', 1, 'Warning signal ID')
      | port_label('input', 2, 'Maneuvre ID')
      | port_label('input', 3, 'Vehicle Type')
      | port_label('input', 4, 'Braking Capacity')
      | port_label('input', 5, 'Vehicle Size')
      | port_label('input', 6, 'spare')
  CAM generation time step : Constant  Value=0.1
  CAM repetition time step : Constant  Value=0.05
  Cartesian to GPS : Subsystem
  Green light : Constant  Value=0
  Ground_{1..5} (5 alike) : Ground
  Infrastructure CAM generator : Subsystem
  Orange light : Constant  Value=0
  Red light : Constant  Value=0
  X : Constant  Value=123.45386505126953
  Y : Constant  Value=85.85821533203125
  Z : Constant  Value=0
  actorID : Constant  Value=7
  actorType : Constant  Value=14
  messageID : Constant  Value=901
  rotZ : Constant  Value=0
  warning : Constant  Value=0
  Antenna_Tx_8 : Outport  Tag=AntennaTOutport
  wires:
  CAM generation time step/1 -> Infrastructure CAM generator/1(CAM gen time step)
  CAM repetition time step/1 -> Infrastructure CAM generator/2(CAM rep time step)
  warning/1 -> Infrastructure CAM generator/3(warning)
  messageID/1 -> Infrastructure CAM generator/4(messageID)
  actorID/1 -> Infrastructure CAM generator/5(actorID)
  actorType/1 -> Infrastructure CAM generator/6(actorType)
  Red light/1 -> Infrastructure CAM generator/7(Red light)
  Orange light/1 -> Infrastructure CAM generator/8(Orange light)
  Green light/1 -> Infrastructure CAM generator/9(Green light)
  rotZ/1 -> Infrastructure CAM generator/13(rotZ)
  Cartesian to GPS/1(lat) -> Infrastructure CAM generator/10(GPS_lat)
  Cartesian to GPS/2(long) -> Infrastructure CAM generator/11(GPS_long)
  Cartesian to GPS/3(alt) -> Infrastructure CAM generator/12(GPS_alt)
  X/1 -> Cartesian to GPS/1(X)
  Y/1 -> Cartesian to GPS/2(Y)
  Z/1 -> Cartesian to GPS/3(Z)
  Antenna_Tx_8_Mux/2(Out2) -> [Antenna_Tx_8]
  [Antenna_Tx_8] -> Antenna_Tx_8_BusAssignment/1
  Antenna_Tx_8_BusAssignment/1 -> Antenna_Tx_8_Mux/6(spare)
  Antenna_Tx_8_Mux/1(Out1) -> Antenna_Tx_8/1
  Infrastructure CAM generator/9(CAM_rotZ) -> Antenna_Tx_8_BusAssignment/10
  Infrastructure CAM generator/8(CAM_GPSalt) -> Antenna_Tx_8_BusAssignment/9
  Infrastructure CAM generator/7(CAM_GPSlong) -> Antenna_Tx_8_BusAssignment/8
  Infrastructure CAM generator/6(CAM_GPSlat) -> Antenna_Tx_8_BusAssignment/7
  Infrastructure CAM generator/5(CAM_trafficLightPriority) -> Antenna_Tx_8_BusAssignment/6
  Infrastructure CAM generator/4(CAM_vehicleType) -> Antenna_Tx_8_BusAssignment/5
  Infrastructure CAM generator/3(CAM_vehicleID) -> Antenna_Tx_8_BusAssignment/4
  Infrastructure CAM generator/2(CAM_messageID) -> Antenna_Tx_8_BusAssignment/3
  Infrastructure CAM generator/1(CAM_warning) -> Antenna_Tx_8_BusAssignment/2
  Ground_1/1 -> Antenna_Tx_8_Mux/1(warning_signal_id)
  Ground_2/1 -> Antenna_Tx_8_Mux/2(maneuvre_id)
  Ground_3/1 -> Antenna_Tx_8_Mux/3(vehicle_type)
  Ground_4/1 -> Antenna_Tx_8_Mux/4(brakingcapacity)
  Ground_5/1 -> Antenna_Tx_8_Mux/5(vehicle_size)

== system V2x_TC_TrafficLights_cs/TrafficSignPoleNL_10 ==
  Antenna_Tx_15_BusAssignment : BusAssignment  Tag=BusAntennaT  AssignedSignals=warning,messageID,actorID,actorType,trafficLightPriority,GPS_lat,GPS_long,GPS_alt,rotZ
  Antenna_Tx_15_Mux : Subsystem  Tag=MuxAntennaT  mask(PreScan Mux Block:){Channel=1, TimeStep=1/100}
      mask prompts: TimeStep "Time Step [s]"
      settings: DeleteFcn=PreScanBlockDeleteFcn;
      mask Display:
      | fprintf('Channel: %d',Channel)
      | port_label('input', 1, 'Warning signal ID')
      | port_label('input', 2, 'Maneuvre ID')
      | port_label('input', 3, 'Vehicle Type')
      | port_label('input', 4, 'Braking Capacity')
      | port_label('input', 5, 'Vehicle Size')
      | port_label('input', 6, 'spare')
  CAM generation time step : Constant  Value=0.1
  CAM repetition time step : Constant  Value=0.05
  Cartesian to GPS : Subsystem
  Green light : Constant  Value=0
  Ground_{1..5} (5 alike) : Ground
  Infrastructure CAM generator : Subsystem
  Orange light : Constant  Value=0
  Red light : Constant  Value=0
  X : Constant  Value=121.87362670898437
  Y : Constant  Value=29.976778030395508
  Z : Constant  Value=0
  actorID : Constant  Value=7
  actorType : Constant  Value=14
  messageID : Constant  Value=1040
  rotZ : Constant  Value=270
  warning : Constant  Value=0
  Antenna_Tx_15 : Outport  Tag=AntennaTOutport
  wires:
  CAM generation time step/1 -> Infrastructure CAM generator/1(CAM gen time step)
  CAM repetition time step/1 -> Infrastructure CAM generator/2(CAM rep time step)
  warning/1 -> Infrastructure CAM generator/3(warning)
  messageID/1 -> Infrastructure CAM generator/4(messageID)
  actorID/1 -> Infrastructure CAM generator/5(actorID)
  actorType/1 -> Infrastructure CAM generator/6(actorType)
  Red light/1 -> Infrastructure CAM generator/7(Red light)
  Orange light/1 -> Infrastructure CAM generator/8(Orange light)
  Green light/1 -> Infrastructure CAM generator/9(Green light)
  rotZ/1 -> Infrastructure CAM generator/13(rotZ)
  Cartesian to GPS/1(lat) -> Infrastructure CAM generator/10(GPS_lat)
  Cartesian to GPS/2(long) -> Infrastructure CAM generator/11(GPS_long)
  Cartesian to GPS/3(alt) -> Infrastructure CAM generator/12(GPS_alt)
  X/1 -> Cartesian to GPS/1(X)
  Y/1 -> Cartesian to GPS/2(Y)
  Z/1 -> Cartesian to GPS/3(Z)
  Antenna_Tx_15_Mux/2(Out2) -> [Antenna_Tx_15]
  [Antenna_Tx_15] -> Antenna_Tx_15_BusAssignment/1
  Antenna_Tx_15_BusAssignment/1 -> Antenna_Tx_15_Mux/6(spare)
  Antenna_Tx_15_Mux/1(Out1) -> Antenna_Tx_15/1
  Infrastructure CAM generator/9(CAM_rotZ) -> Antenna_Tx_15_BusAssignment/10
  Infrastructure CAM generator/8(CAM_GPSalt) -> Antenna_Tx_15_BusAssignment/9
  Infrastructure CAM generator/7(CAM_GPSlong) -> Antenna_Tx_15_BusAssignment/8
  Infrastructure CAM generator/6(CAM_GPSlat) -> Antenna_Tx_15_BusAssignment/7
  Infrastructure CAM generator/5(CAM_trafficLightPriority) -> Antenna_Tx_15_BusAssignment/6
  Infrastructure CAM generator/4(CAM_vehicleType) -> Antenna_Tx_15_BusAssignment/5
  Infrastructure CAM generator/3(CAM_vehicleID) -> Antenna_Tx_15_BusAssignment/4
  Infrastructure CAM generator/2(CAM_messageID) -> Antenna_Tx_15_BusAssignment/3
  Infrastructure CAM generator/1(CAM_warning) -> Antenna_Tx_15_BusAssignment/2
  Ground_1/1 -> Antenna_Tx_15_Mux/1(warning_signal_id)
  Ground_2/1 -> Antenna_Tx_15_Mux/2(maneuvre_id)
  Ground_3/1 -> Antenna_Tx_15_Mux/3(vehicle_type)
  Ground_4/1 -> Antenna_Tx_15_Mux/4(brakingcapacity)
  Ground_5/1 -> Antenna_Tx_15_Mux/5(vehicle_size)
